simulink model "isi_corr"  (Simulink 7.0)
settings: Solver=ode45  StartTime=0.0  StopTime=500  RelTol=1e-3
undefined symbols (not defined in the file): bit_rev, pfb_coeff_gen_calc
libraries: xbsIndex_r4 x4180, casper_library x399, isi_corr_lib x8, xps_library x5, dspsigops x2, simulink x1
(part 2 of 45)

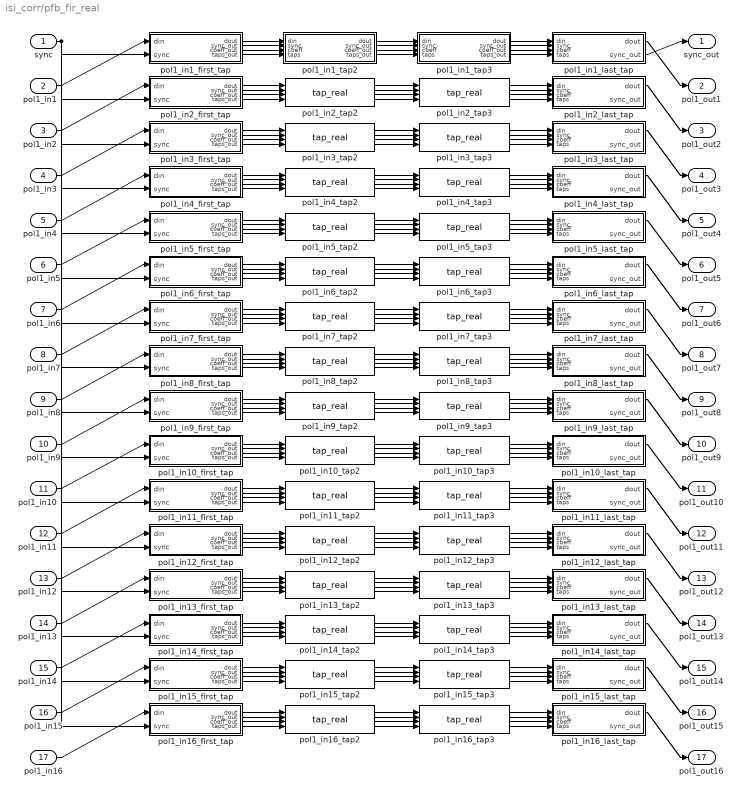
== system isi_corr/pfb_fir_real ==
  sync : Inport  OutDataType=sfix(16)  OutScaling=2^0
  pol1_in1 : Inport  Port=2  OutDataType=sfix(16)  OutScaling=2^0
  pol1_in2 : Inport  Port=3
  pol1_in3 : Inport  Port=4
  pol1_in4 : Inport  Port=5
  pol1_in5 : Inport  Port=6
  pol1_in6 : Inport  Port=7
  pol1_in7 : Inport  Port=8
  pol1_in8 : Inport  Port=9
  pol1_in9 : Inport  Port=10
  pol1_in10 : Inport  Port=11
  pol1_in11 : Inport  Port=12
  pol1_in12 : Inport  Port=13
  pol1_in13 : Inport  Port=14
  pol1_in14 : Inport  Port=15
  pol1_in15 : Inport  Port=16
  pol1_in16 : Inport  Port=17
  pol1_in10_first_tap : Subsystem  AncestorBlock=casper_library/PFBs/first_tap_real  mask(first_tap_real){nput=9, PFBSize=8, CoeffBitWidth=18, TotalTaps=4, BitWidthIn=8, CoeffDistMem=0, WindowType=hamming, mult_latency=2, bram_latency=2, n_inputs=4, fwidth=1}
      mask prompts: nput "This is input number:"; PFBSize "Size of PFB: (2^? pnts)"; CoeffBitWidth "Bitwidth of Coefficients:"; TotalTaps "Total Number of Taps:"; BitWidthIn "Input Bitwidth:"; CoeffDistMem "Implement Coeff Gen in Distributed Memory:"; WindowType "Windowing Function:"; mult_latency "Mult Latency"; bram_latency "BRAM Latency"; n_inputs "Number of Simultaneous Inputs: (2^?)"; fwidth "Bit Width (normal=1)"
      mask init:
      | first_tap_real_init(gcb,...
      |     'nput', nput,...
      |     'PFBSize', PFBSize,...
      |     'CoeffBitWidth', CoeffBitWidth,...
      |     'TotalTaps', TotalTaps,...
      |     'BitWidthIn', BitWidthIn,...
      |     'CoeffDistMem', CoeffDistMem,...
      |     'WindowType', WindowType,...
      |     'mult_latency', mult_latency,...
      |     'bram_latency', bram_latency,...
      |     'n_inputs', n_inputs,...
      |     'fwidth', fwidth);
      settings: AttributesFormatString=input=9, taps=4
      mask StyleString: edit,edit,edit,edit,edit,edit,popup(bartlett|barthannwin|blackman|blackmanharris|bohamwin|chebwin|flattopwin|gausswin|hamming|hann|kaiser|nuttallwin|parzenwin|rectwin|tukeywin|triang|userwindow),edit,edit,edit,edit
  pol1_in10_last_tap : Subsystem  AncestorBlock=casper_library/PFBs/last_tap_real  mask(last_tap_real){TotalTaps=4, BitWidthIn=8, BitWidthOut=18, CoeffBitWidth=18, add_latency=1, mult_latency=2, quantization=Truncate}
      mask prompts: TotalTaps "Total Number of Taps:"; BitWidthIn "Input Bitwidth:"; BitWidthOut "Output Bitwidth:"; CoeffBitWidth "Coeff Bitwidth:"; add_latency "Add Latency"; mult_latency "Mult Latency"; quantization "Quantization Behavior"
      mask init:
      | last_tap_real_init(gcb,...
      |     'TotalTaps', TotalTaps,...
      |     'BitWidthIn', BitWidthIn,...
      |     'BitWidthOut', BitWidthOut,...
      |     'CoeffBitWidth', CoeffBitWidth,...
      |     'add_latency', add_latency,...
      |     'mult_latency', mult_latency,...
      |     'quantization', quantization);
      settings: AttributesFormatString=taps=4
      mask StyleString: edit,edit,edit,edit,edit,edit,popup(Truncate|Round  (unbiased: +/- Inf)|Round  (unbiased: Even Values))
  pol1_in10_tap2 : casper_library/PFBs/tap_real  SystemSampleTime=-1  mult_latency=2  coeff_width=18  coeff_frac_width=17  delay=16  data_width=8  bram_latency=2
  pol1_in10_tap3 : casper_library/PFBs/tap_real  SystemSampleTime=-1  mult_latency=2  coeff_width=18  coeff_frac_width=17  delay=16  data_width=8  bram_latency=2
  pol1_in11_first_tap : Subsystem  AncestorBlock=casper_library/PFBs/first_tap_real  mask(first_tap_real){nput=10, PFBSize=8, CoeffBitWidth=18, TotalTaps=4, BitWidthIn=8, CoeffDistMem=0, WindowType=hamming, mult_latency=2, bram_latency=2, n_inputs=4, fwidth=1}
      mask prompts: nput "This is input number:"; PFBSize "Size of PFB: (2^? pnts)"; CoeffBitWidth "Bitwidth of Coefficients:"; TotalTaps "Total Number of Taps:"; BitWidthIn "Input Bitwidth:"; CoeffDistMem "Implement Coeff Gen in Distributed Memory:"; WindowType "Windowing Function:"; mult_latency "Mult Latency"; bram_latency "BRAM Latency"; n_inputs "Number of Simultaneous Inputs: (2^?)"; fwidth "Bit Width (normal=1)"
      mask init: same as isi_corr/pfb_fir_real/pol1_in10_first_tap
      settings: AttributesFormatString=input=10, taps=4
      mask StyleString: edit,edit,edit,edit,edit,edit,popup(bartlett|barthannwin|blackman|blackmanharris|bohamwin|chebwin|flattopwin|gausswin|hamming|hann|kaiser|nuttallwin|parzenwin|rectwin|tukeywin|triang|userwindow),edit,edit,edit,edit
  pol1_in11_last_tap : Subsystem  AncestorBlock=casper_library/PFBs/last_tap_real  mask(last_tap_real){TotalTaps=4, BitWidthIn=8, BitWidthOut=18, CoeffBitWidth=18, add_latency=1, mult_latency=2, quantization=Truncate}
      mask prompts: TotalTaps "Total Number of Taps:"; BitWidthIn "Input Bitwidth:"; BitWidthOut "Output Bitwidth:"; CoeffBitWidth "Coeff Bitwidth:"; add_latency "Add Latency"; mult_latency "Mult Latency"; quantization "Quantization Behavior"
      mask init: same as isi_corr/pfb_fir_real/pol1_in10_last_tap
      settings: AttributesFormatString=taps=4
      mask StyleString: edit,edit,edit,edit,edit,edit,popup(Truncate|Round  (unbiased: +/- Inf)|Round  (unbiased: Even Values))
  pol1_in11_tap2 : casper_library/PFBs/tap_real  SystemSampleTime=-1  mult_latency=2  coeff_width=18  coeff_frac_width=17  delay=16  data_width=8  bram_latency=2
  pol1_in11_tap3 : casper_library/PFBs/tap_real  SystemSampleTime=-1  mult_latency=2  coeff_width=18  coeff_frac_width=17  delay=16  data_width=8  bram_latency=2
  pol1_in12_first_tap : Subsystem  AncestorBlock=casper_library/PFBs/first_tap_real  mask(first_tap_real){nput=11, PFBSize=8, CoeffBitWidth=18, TotalTaps=4, BitWidthIn=8, CoeffDistMem=0, WindowType=hamming, mult_latency=2, bram_latency=2, n_inputs=4, fwidth=1}
      mask prompts: nput "This is input number:"; PFBSize "Size of PFB: (2^? pnts)"; CoeffBitWidth "Bitwidth of Coefficients:"; TotalTaps "Total Number of Taps:"; BitWidthIn "Input Bitwidth:"; CoeffDistMem "Implement Coeff Gen in Distributed Memory:"; WindowType "Windowing Function:"; mult_latency "Mult Latency"; bram_latency "BRAM Latency"; n_inputs "Number of Simultaneous Inputs: (2^?)"; fwidth "Bit Width (normal=1)"
      mask init: same as isi_corr/pfb_fir_real/pol1_in10_first_tap
      settings: AttributesFormatString=input=11, taps=4
      mask StyleString: edit,edit,edit,edit,edit,edit,popup(bartlett|barthannwin|blackman|blackmanharris|bohamwin|chebwin|flattopwin|gausswin|hamming|hann|kaiser|nuttallwin|parzenwin|rectwin|tukeywin|triang|userwindow),edit,edit,edit,edit
  pol1_in12_last_tap : Subsystem  AncestorBlock=casper_library/PFBs/last_tap_real  mask(last_tap_real){TotalTaps=4, BitWidthIn=8, BitWidthOut=18, CoeffBitWidth=18, add_latency=1, mult_latency=2, quantization=Truncate}
      mask prompts: TotalTaps "Total Number of Taps:"; BitWidthIn "Input Bitwidth:"; BitWidthOut "Output Bitwidth:"; CoeffBitWidth "Coeff Bitwidth:"; add_latency "Add Latency"; mult_latency "Mult Latency"; quantization "Quantization Behavior"
      mask init: same as isi_corr/pfb_fir_real/pol1_in10_last_tap
      settings: AttributesFormatString=taps=4
      mask StyleString: edit,edit,edit,edit,edit,edit,popup(Truncate|Round  (unbiased: +/- Inf)|Round  (unbiased: Even Values))
  pol1_in12_tap2 : casper_library/PFBs/tap_real  SystemSampleTime=-1  mult_latency=2  coeff_width=18  coeff_frac_width=17  delay=16  data_width=8  bram_latency=2
  pol1_in12_tap3 : casper_library/PFBs/tap_real  SystemSampleTime=-1  mult_latency=2  coeff_width=18  coeff_frac_width=17  delay=16  data_width=8  bram_latency=2
  pol1_in13_first_tap : Subsystem  AncestorBlock=casper_library/PFBs/first_tap_real  mask(first_tap_real){nput=12, PFBSize=8, CoeffBitWidth=18, TotalTaps=4, BitWidthIn=8, CoeffDistMem=0, WindowType=hamming, mult_latency=2, bram_latency=2, n_inputs=4, fwidth=1}
      mask prompts: nput "This is input number:"; PFBSize "Size of PFB: (2^? pnts)"; CoeffBitWidth "Bitwidth of Coefficients:"; TotalTaps "Total Number of Taps:"; BitWidthIn "Input Bitwidth:"; CoeffDistMem "Implement Coeff Gen in Distributed Memory:"; WindowType "Windowing Function:"; mult_latency "Mult Latency"; bram_latency "BRAM Latency"; n_inputs "Number of Simultaneous Inputs: (2^?)"; fwidth "Bit Width (normal=1)"
      mask init: same as isi_corr/pfb_fir_real/pol1_in10_first_tap
      settings: AttributesFormatString=input=12, taps=4
      mask StyleString: edit,edit,edit,edit,edit,edit,popup(bartlett|barthannwin|blackman|blackmanharris|bohamwin|chebwin|flattopwin|gausswin|hamming|hann|kaiser|nuttallwin|parzenwin|rectwin|tukeywin|triang|userwindow),edit,edit,edit,edit
  pol1_in13_last_tap : Subsystem  AncestorBlock=casper_library/PFBs/last_tap_real  mask(last_tap_real){TotalTaps=4, BitWidthIn=8, BitWidthOut=18, CoeffBitWidth=18, add_latency=1, mult_latency=2, quantization=Truncate}
      mask prompts: TotalTaps "Total Number of Taps:"; BitWidthIn "Input Bitwidth:"; BitWidthOut "Output Bitwidth:"; CoeffBitWidth "Coeff Bitwidth:"; add_latency "Add Latency"; mult_latency "Mult Latency"; quantization "Quantization Behavior"
      mask init: same as isi_corr/pfb_fir_real/pol1_in10_last_tap
      settings: AttributesFormatString=taps=4
      mask StyleString: edit,edit,edit,edit,edit,edit,popup(Truncate|Round  (unbiased: +/- Inf)|Round  (unbiased: Even Values))
  pol1_in13_tap2 : casper_library/PFBs/tap_real  SystemSampleTime=-1  mult_latency=2  coeff_width=18  coeff_frac_width=17  delay=16  data_width=8  bram_latency=2
  pol1_in13_tap3 : casper_library/PFBs/tap_real  SystemSampleTime=-1  mult_latency=2  coeff_width=18  coeff_frac_width=17  delay=16  data_width=8  bram_latency=2
  pol1_in14_first_tap : Subsystem  AncestorBlock=casper_library/PFBs/first_tap_real  mask(first_tap_real){nput=13, PFBSize=8, CoeffBitWidth=18, TotalTaps=4, BitWidthIn=8, CoeffDistMem=0, WindowType=hamming, mult_latency=2, bram_latency=2, n_inputs=4, fwidth=1}
      mask prompts: nput "This is input number:"; PFBSize "Size of PFB: (2^? pnts)"; CoeffBitWidth "Bitwidth of Coefficients:"; TotalTaps "Total Number of Taps:"; BitWidthIn "Input Bitwidth:"; CoeffDistMem "Implement Coeff Gen in Distributed Memory:"; WindowType "Windowing Function:"; mult_latency "Mult Latency"; bram_latency "BRAM Latency"; n_inputs "Number of Simultaneous Inputs: (2^?)"; fwidth "Bit Width (normal=1)"
      mask init: same as isi_corr/pfb_fir_real/pol1_in10_first_tap
      settings: AttributesFormatString=input=13, taps=4
      mask StyleString: edit,edit,edit,edit,edit,edit,popup(bartlett|barthannwin|blackman|blackmanharris|bohamwin|chebwin|flattopwin|gausswin|hamming|hann|kaiser|nuttallwin|parzenwin|rectwin|tukeywin|triang|userwindow),edit,edit,edit,edit
  pol1_in14_last_tap : Subsystem  AncestorBlock=casper_library/PFBs/last_tap_real  mask(last_tap_real){TotalTaps=4, BitWidthIn=8, BitWidthOut=18, CoeffBitWidth=18, add_latency=1, mult_latency=2, quantization=Truncate}
      mask prompts: TotalTaps "Total Number of Taps:"; BitWidthIn "Input Bitwidth:"; BitWidthOut "Output Bitwidth:"; CoeffBitWidth "Coeff Bitwidth:"; add_latency "Add Latency"; mult_latency "Mult Latency"; quantization "Quantization Behavior"
      mask init: same as isi_corr/pfb_fir_real/pol1_in10_last_tap
      settings: AttributesFormatString=taps=4
      mask StyleString: edit,edit,edit,edit,edit,edit,popup(Truncate|Round  (unbiased: +/- Inf)|Round  (unbiased: Even Values))
  pol1_in14_tap2 : casper_library/PFBs/tap_real  SystemSampleTime=-1  mult_latency=2  coeff_width=18  coeff_frac_width=17  delay=16  data_width=8  bram_latency=2
  pol1_in14_tap3 : casper_library/PFBs/tap_real  SystemSampleTime=-1  mult_latency=2  coeff_width=18  coeff_frac_width=17  delay=16  data_width=8  bram_latency=2
  pol1_in15_first_tap : Subsystem  AncestorBlock=casper_library/PFBs/first_tap_real  mask(first_tap_real){nput=14, PFBSize=8, CoeffBitWidth=18, TotalTaps=4, BitWidthIn=8, CoeffDistMem=0, WindowType=hamming, mult_latency=2, bram_latency=2, n_inputs=4, fwidth=1}
      mask prompts: nput "This is input number:"; PFBSize "Size of PFB: (2^? pnts)"; CoeffBitWidth "Bitwidth of Coefficients:"; TotalTaps "Total Number of Taps:"; BitWidthIn "Input Bitwidth:"; CoeffDistMem "Implement Coeff Gen in Distributed Memory:"; WindowType "Windowing Function:"; mult_latency "Mult Latency"; bram_latency "BRAM Latency"; n_inputs "Number of Simultaneous Inputs: (2^?)"; fwidth "Bit Width (normal=1)"
      mask init: same as isi_corr/pfb_fir_real/pol1_in10_first_tap
      settings: AttributesFormatString=input=14, taps=4
      mask StyleString: edit,edit,edit,edit,edit,edit,popup(bartlett|barthannwin|blackman|blackmanharris|bohamwin|chebwin|flattopwin|gausswin|hamming|hann|kaiser|nuttallwin|parzenwin|rectwin|tukeywin|triang|userwindow),edit,edit,edit,edit
  pol1_in15_last_tap : Subsystem  AncestorBlock=casper_library/PFBs/last_tap_real  mask(last_tap_real){TotalTaps=4, BitWidthIn=8, BitWidthOut=18, CoeffBitWidth=18, add_latency=1, mult_latency=2, quantization=Truncate}
      mask prompts: TotalTaps "Total Number of Taps:"; BitWidthIn "Input Bitwidth:"; BitWidthOut "Output Bitwidth:"; CoeffBitWidth "Coeff Bitwidth:"; add_latency "Add Latency"; mult_latency "Mult Latency"; quantization "Quantization Behavior"
      mask init: same as isi_corr/pfb_fir_real/pol1_in10_last_tap
      settings: AttributesFormatString=taps=4
      mask StyleString: edit,edit,edit,edit,edit,edit,popup(Truncate|Round  (unbiased: +/- Inf)|Round  (unbiased: Even Values))
  pol1_in15_tap2 : casper_library/PFBs/tap_real  SystemSampleTime=-1  mult_latency=2  coeff_width=18  coeff_frac_width=17  delay=16  data_width=8  bram_latency=2
  pol1_in15_tap3 : casper_library/PFBs/tap_real  SystemSampleTime=-1  mult_latency=2  coeff_width=18  coeff_frac_width=17  delay=16  data_width=8  bram_latency=2
  pol1_in16_first_tap : Subsystem  AncestorBlock=casper_library/PFBs/first_tap_real  mask(first_tap_real){nput=15, PFBSize=8, CoeffBitWidth=18, TotalTaps=4, BitWidthIn=8, CoeffDistMem=0, WindowType=hamming, mult_latency=2, bram_latency=2, n_inputs=4, fwidth=1}
      mask prompts: nput "This is input number:"; PFBSize "Size of PFB: (2^? pnts)"; CoeffBitWidth "Bitwidth of Coefficients:"; TotalTaps "Total Number of Taps:"; BitWidthIn "Input Bitwidth:"; CoeffDistMem "Implement Coeff Gen in Distributed Memory:"; WindowType "Windowing Function:"; mult_latency "Mult Latency"; bram_latency "BRAM Latency"; n_inputs "Number of Simultaneous Inputs: (2^?)"; fwidth "Bit Width (normal=1)"
      mask init: same as isi_corr/pfb_fir_real/pol1_in10_first_tap
      settings: AttributesFormatString=input=15, taps=4
      mask StyleString: edit,edit,edit,edit,edit,edit,popup(bartlett|barthannwin|blackman|blackmanharris|bohamwin|chebwin|flattopwin|gausswin|hamming|hann|kaiser|nuttallwin|parzenwin|rectwin|tukeywin|triang|userwindow),edit,edit,edit,edit
  pol1_in16_last_tap : Subsystem  AncestorBlock=casper_library/PFBs/last_tap_real  mask(last_tap_real){TotalTaps=4, BitWidthIn=8, BitWidthOut=18, CoeffBitWidth=18, add_latency=1, mult_latency=2, quantization=Truncate}
      mask prompts: TotalTaps "Total Number of Taps:"; BitWidthIn "Input Bitwidth:"; BitWidthOut "Output Bitwidth:"; CoeffBitWidth "Coeff Bitwidth:"; add_latency "Add Latency"; mult_latency "Mult Latency"; quantization "Quantization Behavior"
      mask init: same as isi_corr/pfb_fir_real/pol1_in10_last_tap
      settings: AttributesFormatString=taps=4
      mask StyleString: edit,edit,edit,edit,edit,edit,popup(Truncate|Round  (unbiased: +/- Inf)|Round  (unbiased: Even Values))
  pol1_in16_tap2 : casper_library/PFBs/tap_real  SystemSampleTime=-1  mult_latency=2  coeff_width=18  coeff_frac_width=17  delay=16  data_width=8  bram_latency=2
  pol1_in16_tap3 : casper_library/PFBs/tap_real  SystemSampleTime=-1  mult_latency=2  coeff_width=18  coeff_frac_width=17  delay=16  data_width=8  bram_latency=2
  pol1_in1_first_tap : Subsystem  AncestorBlock=casper_library/PFBs/first_tap_real  mask(first_tap_real){nput=0, PFBSize=8, CoeffBitWidth=18, TotalTaps=4, BitWidthIn=8, CoeffDistMem=0, WindowType=hamming, mult_latency=2, bram_latency=2, n_inputs=4, fwidth=1}
      mask prompts: nput "This is input number:"; PFBSize "Size of PFB: (2^? pnts)"; CoeffBitWidth "Bitwidth of Coefficients:"; TotalTaps "Total Number of Taps:"; BitWidthIn "Input Bitwidth:"; CoeffDistMem "Implement Coeff Gen in Distributed Memory:"; WindowType "Windowing Function:"; mult_latency "Mult Latency"; bram_latency "BRAM Latency"; n_inputs "Number of Simultaneous Inputs: (2^?)"; fwidth "Bit Width (normal=1)"
      mask init: same as isi_corr/pfb_fir_real/pol1_in10_first_tap
      settings: AttributesFormatString=input=0, taps=4
      mask StyleString: edit,edit,edit,edit,edit,edit,popup(bartlett|barthannwin|blackman|blackmanharris|bohamwin|chebwin|flattopwin|gausswin|hamming|hann|kaiser|nuttallwin|parzenwin|rectwin|tukeywin|triang|userwindow),edit,edit,edit,edit
  pol1_in1_last_tap : Subsystem  AncestorBlock=casper_library/PFBs/last_tap_real  mask(last_tap_real){TotalTaps=4, BitWidthIn=8, BitWidthOut=18, CoeffBitWidth=18, add_latency=1, mult_latency=2, quantization=Truncate}
      mask prompts: TotalTaps "Total Number of Taps:"; BitWidthIn "Input Bitwidth:"; BitWidthOut "Output Bitwidth:"; CoeffBitWidth "Coeff Bitwidth:"; add_latency "Add Latency"; mult_latency "Mult Latency"; quantization "Quantization Behavior"
      mask init: same as isi_corr/pfb_fir_real/pol1_in10_last_tap
      settings: AttributesFormatString=taps=4
      mask StyleString: edit,edit,edit,edit,edit,edit,popup(Truncate|Round  (unbiased: +/- Inf)|Round  (unbiased: Even Values))
  pol1_in1_tap2 : Subsystem  AncestorBlock=casper_library/PFBs/tap_real  mask(pfb_tap_real){mult_latency=2, coeff_width=18, coeff_frac_width=17, delay=16, data_width=8, bram_latency=2}
      mask prompts: mult_latency "Multiplier Latency"; coeff_width "Coefficient Bit Width In"; coeff_frac_width "Coefficient Fractional Bits"; delay "Data/Sync Delay"; data_width "Data Bit Width In"; bram_latency "BRAM Latency"
      mask init:
      | cursys = gcb;
      | disp(cursys);
  pol1_in1_tap3 : Subsystem  AncestorBlock=casper_library/PFBs/tap_real  mask(pfb_tap_real){mult_latency=2, coeff_width=18, coeff_frac_width=17, delay=16, data_width=8, bram_latency=2}
      mask prompts: mult_latency "Multiplier Latency"; coeff_width "Coefficient Bit Width In"; coeff_frac_width "Coefficient Fractional Bits"; delay "Data/Sync Delay"; data_width "Data Bit Width In"; bram_latency "BRAM Latency"
      mask init:
      | cursys = gcb;
      | disp(cursys);
  pol1_in2_first_tap : Subsystem  AncestorBlock=casper_library/PFBs/first_tap_real  mask(first_tap_real){nput=1, PFBSize=8, CoeffBitWidth=18, TotalTaps=4, BitWidthIn=8, CoeffDistMem=0, WindowType=hamming, mult_latency=2, bram_latency=2, n_inputs=4, fwidth=1}
      mask prompts: nput "This is input number:"; PFBSize "Size of PFB: (2^? pnts)"; CoeffBitWidth "Bitwidth of Coefficients:"; TotalTaps "Total Number of Taps:"; BitWidthIn "Input Bitwidth:"; CoeffDistMem "Implement Coeff Gen in Distributed Memory:"; WindowType "Windowing Function:"; mult_latency "Mult Latency"; bram_latency "BRAM Latency"; n_inputs "Number of Simultaneous Inputs: (2^?)"; fwidth "Bit Width (normal=1)"
      mask init: same as isi_corr/pfb_fir_real/pol1_in10_first_tap
      settings: AttributesFormatString=input=1, taps=4
      mask StyleString: edit,edit,edit,edit,edit,edit,popup(bartlett|barthannwin|blackman|blackmanharris|bohamwin|chebwin|flattopwin|gausswin|hamming|hann|kaiser|nuttallwin|parzenwin|rectwin|tukeywin|triang|userwindow),edit,edit,edit,edit
  pol1_in2_last_tap : Subsystem  AncestorBlock=casper_library/PFBs/last_tap_real  mask(last_tap_real){TotalTaps=4, BitWidthIn=8, BitWidthOut=18, CoeffBitWidth=18, add_latency=1, mult_latency=2, quantization=Truncate}
      mask prompts: TotalTaps "Total Number of Taps:"; BitWidthIn "Input Bitwidth:"; BitWidthOut "Output Bitwidth:"; CoeffBitWidth "Coeff Bitwidth:"; add_latency "Add Latency"; mult_latency "Mult Latency"; quantization "Quantization Behavior"
      mask init: same as isi_corr/pfb_fir_real/pol1_in10_last_tap
      settings: AttributesFormatString=taps=4
      mask StyleString: edit,edit,edit,edit,edit,edit,popup(Truncate|Round  (unbiased: +/- Inf)|Round  (unbiased: Even Values))
  pol1_in2_tap2 : casper_library/PFBs/tap_real  SystemSampleTime=-1  mult_latency=2  coeff_width=18  coeff_frac_width=17  delay=16  data_width=8  bram_latency=2
  pol1_in2_tap3 : casper_library/PFBs/tap_real  SystemSampleTime=-1  mult_latency=2  coeff_width=18  coeff_frac_width=17  delay=16  data_width=8  bram_latency=2
  pol1_in3_first_tap : Subsystem  AncestorBlock=casper_library/PFBs/first_tap_real  mask(first_tap_real){nput=2, PFBSize=8, CoeffBitWidth=18, TotalTaps=4, BitWidthIn=8, CoeffDistMem=0, WindowType=hamming, mult_latency=2, bram_latency=2, n_inputs=4, fwidth=1}
      mask prompts: nput "This is input number:"; PFBSize "Size of PFB: (2^? pnts)"; CoeffBitWidth "Bitwidth of Coefficients:"; TotalTaps "Total Number of Taps:"; BitWidthIn "Input Bitwidth:"; CoeffDistMem "Implement Coeff Gen in Distributed Memory:"; WindowType "Windowing Function:"; mult_latency "Mult Latency"; bram_latency "BRAM Latency"; n_inputs "Number of Simultaneous Inputs: (2^?)"; fwidth "Bit Width (normal=1)"
      mask init: same as isi_corr/pfb_fir_real/pol1_in10_first_tap
      settings: AttributesFormatString=input=2, taps=4
      mask StyleString: edit,edit,edit,edit,edit,edit,popup(bartlett|barthannwin|blackman|blackmanharris|bohamwin|chebwin|flattopwin|gausswin|hamming|hann|kaiser|nuttallwin|parzenwin|rectwin|tukeywin|triang|userwindow),edit,edit,edit,edit
  pol1_in3_last_tap : Subsystem  AncestorBlock=casper_library/PFBs/last_tap_real  mask(last_tap_real){TotalTaps=4, BitWidthIn=8, BitWidthOut=18, CoeffBitWidth=18, add_latency=1, mult_latency=2, quantization=Truncate}
      mask prompts: TotalTaps "Total Number of Taps:"; BitWidthIn "Input Bitwidth:"; BitWidthOut "Output Bitwidth:"; CoeffBitWidth "Coeff Bitwidth:"; add_latency "Add Latency"; mult_latency "Mult Latency"; quantization "Quantization Behavior"
      mask init: same as isi_corr/pfb_fir_real/pol1_in10_last_tap
      settings: AttributesFormatString=taps=4
      mask StyleString: edit,edit,edit,edit,edit,edit,popup(Truncate|Round  (unbiased: +/- Inf)|Round  (unbiased: Even Values))
  pol1_in3_tap2 : casper_library/PFBs/tap_real  SystemSampleTime=-1  mult_latency=2  coeff_width=18  coeff_frac_width=17  delay=16  data_width=8  bram_latency=2
  pol1_in3_tap3 : casper_library/PFBs/tap_real  SystemSampleTime=-1  mult_latency=2  coeff_width=18  coeff_frac_width=17  delay=16  data_width=8  bram_latency=2
  pol1_in4_first_tap : Subsystem  AncestorBlock=casper_library/PFBs/first_tap_real  mask(first_tap_real){nput=3, PFBSize=8, CoeffBitWidth=18, TotalTaps=4, BitWidthIn=8, CoeffDistMem=0, WindowType=hamming, mult_latency=2, bram_latency=2, n_inputs=4, fwidth=1}
      mask prompts: nput "This is input number:"; PFBSize "Size of PFB: (2^? pnts)"; CoeffBitWidth "Bitwidth of Coefficients:"; TotalTaps "Total Number of Taps:"; BitWidthIn "Input Bitwidth:"; CoeffDistMem "Implement Coeff Gen in Distributed Memory:"; WindowType "Windowing Function:"; mult_latency "Mult Latency"; bram_latency "BRAM Latency"; n_inputs "Number of Simultaneous Inputs: (2^?)"; fwidth "Bit Width (normal=1)"
      mask init: same as isi_corr/pfb_fir_real/pol1_in10_first_tap
      settings: AttributesFormatString=input=3, taps=4
      mask StyleString: edit,edit,edit,edit,edit,edit,popup(bartlett|barthannwin|blackman|blackmanharris|bohamwin|chebwin|flattopwin|gausswin|hamming|hann|kaiser|nuttallwin|parzenwin|rectwin|tukeywin|triang|userwindow),edit,edit,edit,edit
  pol1_in4_last_tap : Subsystem  AncestorBlock=casper_library/PFBs/last_tap_real  mask(last_tap_real){TotalTaps=4, BitWidthIn=8, BitWidthOut=18, CoeffBitWidth=18, add_latency=1, mult_latency=2, quantization=Truncate}
      mask prompts: TotalTaps "Total Number of Taps:"; BitWidthIn "Input Bitwidth:"; BitWidthOut "Output Bitwidth:"; CoeffBitWidth "Coeff Bitwidth:"; add_latency "Add Latency"; mult_latency "Mult Latency"; quantization "Quantization Behavior"
      mask init: same as isi_corr/pfb_fir_real/pol1_in10_last_tap
      settings: AttributesFormatString=taps=4
      mask StyleString: edit,edit,edit,edit,edit,edit,popup(Truncate|Round  (unbiased: +/- Inf)|Round  (unbiased: Even Values))
  pol1_in4_tap2 : casper_library/PFBs/tap_real  SystemSampleTime=-1  mult_latency=2  coeff_width=18  coeff_frac_width=17  delay=16  data_width=8  bram_latency=2
  pol1_in4_tap3 : casper_library/PFBs/tap_real  SystemSampleTime=-1  mult_latency=2  coeff_width=18  coeff_frac_width=17  delay=16  data_width=8  bram_latency=2
  pol1_in5_first_tap : Subsystem  AncestorBlock=casper_library/PFBs/first_tap_real  mask(first_tap_real){nput=4, PFBSize=8, CoeffBitWidth=18, TotalTaps=4, BitWidthIn=8, CoeffDistMem=0, WindowType=hamming, mult_latency=2, bram_latency=2, n_inputs=4, fwidth=1}
      mask prompts: nput "This is input number:"; PFBSize "Size of PFB: (2^? pnts)"; CoeffBitWidth "Bitwidth of Coefficients:"; TotalTaps "Total Number of Taps:"; BitWidthIn "Input Bitwidth:"; CoeffDistMem "Implement Coeff Gen in Distributed Memory:"; WindowType "Windowing Function:"; mult_latency "Mult Latency"; bram_latency "BRAM Latency"; n_inputs "Number of Simultaneous Inputs: (2^?)"; fwidth "Bit Width (normal=1)"
      mask init: same as isi_corr/pfb_fir_real/pol1_in10_first_tap
      settings: AttributesFormatString=input=4, taps=4
      mask StyleString: edit,edit,edit,edit,edit,edit,popup(bartlett|barthannwin|blackman|blackmanharris|bohamwin|chebwin|flattopwin|gausswin|hamming|hann|kaiser|nuttallwin|parzenwin|rectwin|tukeywin|triang|userwindow),edit,edit,edit,edit
  pol1_in5_last_tap : Subsystem  AncestorBlock=casper_library/PFBs/last_tap_real  mask(last_tap_real){TotalTaps=4, BitWidthIn=8, BitWidthOut=18, CoeffBitWidth=18, add_latency=1, mult_latency=2, quantization=Truncate}
      mask prompts: TotalTaps "Total Number of Taps:"; BitWidthIn "Input Bitwidth:"; BitWidthOut "Output Bitwidth:"; CoeffBitWidth "Coeff Bitwidth:"; add_latency "Add Latency"; mult_latency "Mult Latency"; quantization "Quantization Behavior"
      mask init: same as isi_corr/pfb_fir_real/pol1_in10_last_tap
      settings: AttributesFormatString=taps=4
      mask StyleString: edit,edit,edit,edit,edit,edit,popup(Truncate|Round  (unbiased: +/- Inf)|Round  (unbiased: Even Values))
  pol1_in5_tap2 : casper_library/PFBs/tap_real  SystemSampleTime=-1  mult_latency=2  coeff_width=18  coeff_frac_width=17  delay=16  data_width=8  bram_latency=2
  pol1_in5_tap3 : casper_library/PFBs/tap_real  SystemSampleTime=-1  mult_latency=2  coeff_width=18  coeff_frac_width=17  delay=16  data_width=8  bram_latency=2
  pol1_in6_first_tap : Subsystem  AncestorBlock=casper_library/PFBs/first_tap_real  mask(first_tap_real){nput=5, PFBSize=8, CoeffBitWidth=18, TotalTaps=4, BitWidthIn=8, CoeffDistMem=0, WindowType=hamming, mult_latency=2, bram_latency=2, n_inputs=4, fwidth=1}
      mask prompts: nput "This is input number:"; PFBSize "Size of PFB: (2^? pnts)"; CoeffBitWidth "Bitwidth of Coefficients:"; TotalTaps "Total Number of Taps:"; BitWidthIn "Input Bitwidth:"; CoeffDistMem "Implement Coeff Gen in Distributed Memory:"; WindowType "Windowing Function:"; mult_latency "Mult Latency"; bram_latency "BRAM Latency"; n_inputs "Number of Simultaneous Inputs: (2^?)"; fwidth "Bit Width (normal=1)"
      mask init: same as isi_corr/pfb_fir_real/pol1_in10_first_tap
      settings: AttributesFormatString=input=5, taps=4
      mask StyleString: edit,edit,edit,edit,edit,edit,popup(bartlett|barthannwin|blackman|blackmanharris|bohamwin|chebwin|flattopwin|gausswin|hamming|hann|kaiser|nuttallwin|parzenwin|rectwin|tukeywin|triang|userwindow),edit,edit,edit,edit
  pol1_in6_last_tap : Subsystem  AncestorBlock=casper_library/PFBs/last_tap_real  mask(last_tap_real){TotalTaps=4, BitWidthIn=8, BitWidthOut=18, CoeffBitWidth=18, add_latency=1, mult_latency=2, quantization=Truncate}
      mask prompts: TotalTaps "Total Number of Taps:"; BitWidthIn "Input Bitwidth:"; BitWidthOut "Output Bitwidth:"; CoeffBitWidth "Coeff Bitwidth:"; add_latency "Add Latency"; mult_latency "Mult Latency"; quantization "Quantization Behavior"
      mask init: same as isi_corr/pfb_fir_real/pol1_in10_last_tap
      settings: AttributesFormatString=taps=4
      mask StyleString: edit,edit,edit,edit,edit,edit,popup(Truncate|Round  (unbiased: +/- Inf)|Round  (unbiased: Even Values))
  pol1_in6_tap2 : casper_library/PFBs/tap_real  SystemSampleTime=-1  mult_latency=2  coeff_width=18  coeff_frac_width=17  delay=16  data_width=8  bram_latency=2
  pol1_in6_tap3 : casper_library/PFBs/tap_real  SystemSampleTime=-1  mult_latency=2  coeff_width=18  coeff_frac_width=17  delay=16  data_width=8  bram_latency=2
  pol1_in7_first_tap : Subsystem  AncestorBlock=casper_library/PFBs/first_tap_real  mask(first_tap_real){nput=6, PFBSize=8, CoeffBitWidth=18, TotalTaps=4, BitWidthIn=8, CoeffDistMem=0, WindowType=hamming, mult_latency=2, bram_latency=2, n_inputs=4, fwidth=1}
      mask prompts: nput "This is input number:"; PFBSize "Size of PFB: (2^? pnts)"; CoeffBitWidth "Bitwidth of Coefficients:"; TotalTaps "Total Number of Taps:"; BitWidthIn "Input Bitwidth:"; CoeffDistMem "Implement Coeff Gen in Distributed Memory:"; WindowType "Windowing Function:"; mult_latency "Mult Latency"; bram_latency "BRAM Latency"; n_inputs "Number of Simultaneous Inputs: (2^?)"; fwidth "Bit Width (normal=1)"
      mask init: same as isi_corr/pfb_fir_real/pol1_in10_first_tap
      settings: AttributesFormatString=input=6, taps=4
      mask StyleString: edit,edit,edit,edit,edit,edit,popup(bartlett|barthannwin|blackman|blackmanharris|bohamwin|chebwin|flattopwin|gausswin|hamming|hann|kaiser|nuttallwin|parzenwin|rectwin|tukeywin|triang|userwindow),edit,edit,edit,edit
  pol1_in7_last_tap : Subsystem  AncestorBlock=casper_library/PFBs/last_tap_real  mask(last_tap_real){TotalTaps=4, BitWidthIn=8, BitWidthOut=18, CoeffBitWidth=18, add_latency=1, mult_latency=2, quantization=Truncate}
      mask prompts: TotalTaps "Total Number of Taps:"; BitWidthIn "Input Bitwidth:"; BitWidthOut "Output Bitwidth:"; CoeffBitWidth "Coeff Bitwidth:"; add_latency "Add Latency"; mult_latency "Mult Latency"; quantization "Quantization Behavior"
      mask init: same as isi_corr/pfb_fir_real/pol1_in10_last_tap
      settings: AttributesFormatString=taps=4
      mask StyleString: edit,edit,edit,edit,edit,edit,popup(Truncate|Round  (unbiased: +/- Inf)|Round  (unbiased: Even Values))
  pol1_in7_tap2 : casper_library/PFBs/tap_real  SystemSampleTime=-1  mult_latency=2  coeff_width=18  coeff_frac_width=17  delay=16  data_width=8  bram_latency=2
  pol1_in7_tap3 : casper_library/PFBs/tap_real  SystemSampleTime=-1  mult_latency=2  coeff_width=18  coeff_frac_width=17  delay=16  data_width=8  bram_latency=2
  pol1_in8_first_tap : Subsystem  AncestorBlock=casper_library/PFBs/first_tap_real  mask(first_tap_real){nput=7, PFBSize=8, CoeffBitWidth=18, TotalTaps=4, BitWidthIn=8, CoeffDistMem=0, WindowType=hamming, mult_latency=2, bram_latency=2, n_inputs=4, fwidth=1}
      mask prompts: nput "This is input number:"; PFBSize "Size of PFB: (2^? pnts)"; CoeffBitWidth "Bitwidth of Coefficients:"; TotalTaps "Total Number of Taps:"; BitWidthIn "Input Bitwidth:"; CoeffDistMem "Implement Coeff Gen in Distributed Memory:"; WindowType "Windowing Function:"; mult_latency "Mult Latency"; bram_latency "BRAM Latency"; n_inputs "Number of Simultaneous Inputs: (2^?)"; fwidth "Bit Width (normal=1)"
      mask init: same as isi_corr/pfb_fir_real/pol1_in10_first_tap
      settings: AttributesFormatString=input=7, taps=4
      mask StyleString: edit,edit,edit,edit,edit,edit,popup(bartlett|barthannwin|blackman|blackmanharris|bohamwin|chebwin|flattopwin|gausswin|hamming|hann|kaiser|nuttallwin|parzenwin|rectwin|tukeywin|triang|userwindow),edit,edit,edit,edit
  pol1_in8_last_tap : Subsystem  AncestorBlock=casper_library/PFBs/last_tap_real  mask(last_tap_real){TotalTaps=4, BitWidthIn=8, BitWidthOut=18, CoeffBitWidth=18, add_latency=1, mult_latency=2, quantization=Truncate}
      mask prompts: TotalTaps "Total Number of Taps:"; BitWidthIn "Input Bitwidth:"; BitWidthOut "Output Bitwidth:"; CoeffBitWidth "Coeff Bitwidth:"; add_latency "Add Latency"; mult_latency "Mult Latency"; quantization "Quantization Behavior"
      mask init: same as isi_corr/pfb_fir_real/pol1_in10_last_tap
      settings: AttributesFormatString=taps=4
      mask StyleString: edit,edit,edit,edit,edit,edit,popup(Truncate|Round  (unbiased: +/- Inf)|Round  (unbiased: Even Values))
  pol1_in8_tap2 : casper_library/PFBs/tap_real  SystemSampleTime=-1  mult_latency=2  coeff_width=18  coeff_frac_width=17  delay=16  data_width=8  bram_latency=2
  pol1_in8_tap3 : casper_library/PFBs/tap_real  SystemSampleTime=-1  mult_latency=2  coeff_width=18  coeff_frac_width=17  delay=16  data_width=8  bram_latency=2
  pol1_in9_first_tap : Subsystem  AncestorBlock=casper_library/PFBs/first_tap_real  mask(first_tap_real){nput=8, PFBSize=8, CoeffBitWidth=18, TotalTaps=4, BitWidthIn=8, CoeffDistMem=0, WindowType=hamming, mult_latency=2, bram_latency=2, n_inputs=4, fwidth=1}
      mask prompts: nput "This is input number:"; PFBSize "Size of PFB: (2^? pnts)"; CoeffBitWidth "Bitwidth of Coefficients:"; TotalTaps "Total Number of Taps:"; BitWidthIn "Input Bitwidth:"; CoeffDistMem "Implement Coeff Gen in Distributed Memory:"; WindowType "Windowing Function:"; mult_latency "Mult Latency"; bram_latency "BRAM Latency"; n_inputs "Number of Simultaneous Inputs: (2^?)"; fwidth "Bit Width (normal=1)"
      mask init: same as isi_corr/pfb_fir_real/pol1_in10_first_tap
      settings: AttributesFormatString=input=8, taps=4
      mask StyleString: edit,edit,edit,edit,edit,edit,popup(bartlett|barthannwin|blackman|blackmanharris|bohamwin|chebwin|flattopwin|gausswin|hamming|hann|kaiser|nuttallwin|parzenwin|rectwin|tukeywin|triang|userwindow),edit,edit,edit,edit
  pol1_in9_last_tap : Subsystem  AncestorBlock=casper_library/PFBs/last_tap_real  mask(last_tap_real){TotalTaps=4, BitWidthIn=8, BitWidthOut=18, CoeffBitWidth=18, add_latency=1, mult_latency=2, quantization=Truncate}
      mask prompts: TotalTaps "Total Number of Taps:"; BitWidthIn "Input Bitwidth:"; BitWidthOut "Output Bitwidth:"; CoeffBitWidth "Coeff Bitwidth:"; add_latency "Add Latency"; mult_latency "Mult Latency"; quantization "Quantization Behavior"
      mask init: same as isi_corr/pfb_fir_real/pol1_in10_last_tap
      settings: AttributesFormatString=taps=4
      mask StyleString: edit,edit,edit,edit,edit,edit,popup(Truncate|Round  (unbiased: +/- Inf)|Round  (unbiased: Even Values))
  pol1_in9_tap2 : casper_library/PFBs/tap_real  SystemSampleTime=-1  mult_latency=2  coeff_width=18  coeff_frac_width=17  delay=16  data_width=8  bram_latency=2
  pol1_in9_tap3 : casper_library/PFBs/tap_real  SystemSampleTime=-1  mult_latency=2  coeff_width=18  coeff_frac_width=17  delay=16  data_width=8  bram_latency=2
  sync_out : Outport  OutDataType=sfix(16)  OutScaling=2^0
  pol1_out1 : Outport  Port=2  OutDataType=sfix(16)  OutScaling=2^0
  pol1_out2 : Outport  Port=3
  pol1_out3 : Outport  Port=4
  pol1_out4 : Outport  Port=5
  pol1_out5 : Outport  Port=6
  pol1_out6 : Outport  Port=7
  pol1_out7 : Outport  Port=8
  pol1_out8 : Outport  Port=9
  pol1_out9 : Outport  Port=10
  pol1_out10 : Outport  Port=11
  pol1_out11 : Outport  Port=12
  pol1_out12 : Outport  Port=13
  pol1_out13 : Outport  Port=14
  pol1_out14 : Outport  Port=15
  pol1_out15 : Outport  Port=16
  pol1_out16 : Outport  Port=17
  wires:
  pol1_in1/1 -> pol1_in1_first_tap/1(din)
  sync/1 -> pol1_in1_first_tap/2(sync), pol1_in2_first_tap/2(sync), pol1_in3_first_tap/2(sync), pol1_in4_first_tap/2(sync), pol1_in5_first_tap/2(sync), pol1_in6_first_tap/2(sync), pol1_in7_first_tap/2(sync), pol1_in8_first_tap/2(sync), pol1_in9_first_tap/2(sync), pol1_in10_first_tap/2(sync), pol1_in11_first_tap/2(sync), pol1_in12_first_tap/2(sync), pol1_in13_first_tap/2(sync), pol1_in14_first_tap/2(sync), pol1_in15_first_tap/2(sync), pol1_in16_first_tap/2(sync)
  pol1_in1_first_tap/1(dout) -> pol1_in1_tap2/1(din)
  pol1_in1_first_tap/2(sync_out) -> pol1_in1_tap2/2(sync)
  pol1_in1_first_tap/3(coeff_out) -> pol1_in1_tap2/3(coeff)
  pol1_in1_first_tap/4(taps_out) -> pol1_in1_tap2/4(taps)
  pol1_in1_tap2/1(dout) -> pol1_in1_tap3/1(din)
  pol1_in1_tap2/2(sync_out) -> pol1_in1_tap3/2(sync)
  pol1_in1_tap2/3(coeff_out) -> pol1_in1_tap3/3(coeff)
  pol1_in1_tap2/4(taps_out) -> pol1_in1_tap3/4(taps)
  pol1_in1_tap3/1(dout) -> pol1_in1_last_tap/1(din)
  pol1_in1_tap3/2(sync_out) -> pol1_in1_last_tap/2(sync)
  pol1_in1_tap3/3(coeff_out) -> pol1_in1_last_tap/3(coeff)
  pol1_in1_tap3/4(taps_out) -> pol1_in1_last_tap/4(taps)
  pol1_in1_last_tap/1(dout) -> pol1_out1/1
  pol1_in1_last_tap/2(sync_out) -> sync_out/1
  pol1_in2/1 -> pol1_in2_first_tap/1(din)
  pol1_in2_first_tap/1(dout) -> pol1_in2_tap2/1
  pol1_in2_first_tap/2(sync_out) -> pol1_in2_tap2/2
  pol1_in2_first_tap/3(coeff_out) -> pol1_in2_tap2/3
  pol1_in2_first_tap/4(taps_out) -> pol1_in2_tap2/4
  pol1_in2_tap2/1 -> pol1_in2_tap3/1
  pol1_in2_tap2/2 -> pol1_in2_tap3/2
  pol1_in2_tap2/3 -> pol1_in2_tap3/3
  pol1_in2_tap2/4 -> pol1_in2_tap3/4
  pol1_in2_tap3/1 -> pol1_in2_last_tap/1(din)
  pol1_in2_tap3/2 -> pol1_in2_last_tap/2(sync)
  pol1_in2_tap3/3 -> pol1_in2_last_tap/3(coeff)
  pol1_in2_tap3/4 -> pol1_in2_last_tap/4(taps)
  pol1_in2_last_tap/1(dout) -> pol1_out2/1
  pol1_in3/1 -> pol1_in3_first_tap/1(din)
  pol1_in3_first_tap/1(dout) -> pol1_in3_tap2/1
  pol1_in3_first_tap/2(sync_out) -> pol1_in3_tap2/2
  pol1_in3_first_tap/3(coeff_out) -> pol1_in3_tap2/3
  pol1_in3_first_tap/4(taps_out) -> pol1_in3_tap2/4
  pol1_in3_tap2/1 -> pol1_in3_tap3/1
  pol1_in3_tap2/2 -> pol1_in3_tap3/2
  pol1_in3_tap2/3 -> pol1_in3_tap3/3
  pol1_in3_tap2/4 -> pol1_in3_tap3/4
  pol1_in3_tap3/1 -> pol1_in3_last_tap/1(din)
  pol1_in3_tap3/2 -> pol1_in3_last_tap/2(sync)
  pol1_in3_tap3/3 -> pol1_in3_last_tap/3(coeff)
  pol1_in3_tap3/4 -> pol1_in3_last_tap/4(taps)
  pol1_in3_last_tap/1(dout) -> pol1_out3/1
  pol1_in4/1 -> pol1_in4_first_tap/1(din)
  pol1_in4_first_tap/1(dout) -> pol1_in4_tap2/1
  pol1_in4_first_tap/2(sync_out) -> pol1_in4_tap2/2
  pol1_in4_first_tap/3(coeff_out) -> pol1_in4_tap2/3
  pol1_in4_first_tap/4(taps_out) -> pol1_in4_tap2/4
  pol1_in4_tap2/1 -> pol1_in4_tap3/1
  pol1_in4_tap2/2 -> pol1_in4_tap3/2
  pol1_in4_tap2/3 -> pol1_in4_tap3/3
  pol1_in4_tap2/4 -> pol1_in4_tap3/4
  pol1_in4_tap3/1 -> pol1_in4_last_tap/1(din)
  pol1_in4_tap3/2 -> pol1_in4_last_tap/2(sync)
  pol1_in4_tap3/3 -> pol1_in4_last_tap/3(coeff)
  pol1_in4_tap3/4 -> pol1_in4_last_tap/4(taps)
  pol1_in4_last_tap/1(dout) -> pol1_out4/1
  pol1_in5/1 -> pol1_in5_first_tap/1(din)
  pol1_in5_first_tap/1(dout) -> pol1_in5_tap2/1
  pol1_in5_first_tap/2(sync_out) -> pol1_in5_tap2/2
  pol1_in5_first_tap/3(coeff_out) -> pol1_in5_tap2/3
  pol1_in5_first_tap/4(taps_out) -> pol1_in5_tap2/4
  pol1_in5_tap2/1 -> pol1_in5_tap3/1
  pol1_in5_tap2/2 -> pol1_in5_tap3/2
  pol1_in5_tap2/3 -> pol1_in5_tap3/3
  pol1_in5_tap2/4 -> pol1_in5_tap3/4
  pol1_in5_tap3/1 -> pol1_in5_last_tap/1(din)
  pol1_in5_tap3/2 -> pol1_in5_last_tap/2(sync)
  pol1_in5_tap3/3 -> pol1_in5_last_tap/3(coeff)
  pol1_in5_tap3/4 -> pol1_in5_last_tap/4(taps)
  pol1_in5_last_tap/1(dout) -> pol1_out5/1
  pol1_in6/1 -> pol1_in6_first_tap/1(din)
  pol1_in6_first_tap/1(dout) -> pol1_in6_tap2/1
  pol1_in6_first_tap/2(sync_out) -> pol1_in6_tap2/2
  pol1_in6_first_tap/3(coeff_out) -> pol1_in6_tap2/3
  pol1_in6_first_tap/4(taps_out) -> pol1_in6_tap2/4
  pol1_in6_tap2/1 -> pol1_in6_tap3/1
  pol1_in6_tap2/2 -> pol1_in6_tap3/2
  pol1_in6_tap2/3 -> pol1_in6_tap3/3
  pol1_in6_tap2/4 -> pol1_in6_tap3/4
  pol1_in6_tap3/1 -> pol1_in6_last_tap/1(din)
  pol1_in6_tap3/2 -> pol1_in6_last_tap/2(sync)
  pol1_in6_tap3/3 -> pol1_in6_last_tap/3(coeff)
  pol1_in6_tap3/4 -> pol1_in6_last_tap/4(taps)
  pol1_in6_last_tap/1(dout) -> pol1_out6/1
  pol1_in7/1 -> pol1_in7_first_tap/1(din)
  pol1_in7_first_tap/1(dout) -> pol1_in7_tap2/1
  pol1_in7_first_tap/2(sync_out) -> pol1_in7_tap2/2
  pol1_in7_first_tap/3(coeff_out) -> pol1_in7_tap2/3
  pol1_in7_first_tap/4(taps_out) -> pol1_in7_tap2/4
  pol1_in7_tap2/1 -> pol1_in7_tap3/1
  pol1_in7_tap2/2 -> pol1_in7_tap3/2
  pol1_in7_tap2/3 -> pol1_in7_tap3/3
  pol1_in7_tap2/4 -> pol1_in7_tap3/4
  pol1_in7_tap3/1 -> pol1_in7_last_tap/1(din)
  pol1_in7_tap3/2 -> pol1_in7_last_tap/2(sync)
  pol1_in7_tap3/3 -> pol1_in7_last_tap/3(coeff)
  pol1_in7_tap3/4 -> pol1_in7_last_tap/4(taps)
  pol1_in7_last_tap/1(dout) -> pol1_out7/1
  pol1_in8/1 -> pol1_in8_first_tap/1(din)
  pol1_in8_first_tap/1(dout) -> pol1_in8_tap2/1
  pol1_in8_first_tap/2(sync_out) -> pol1_in8_tap2/2
  pol1_in8_first_tap/3(coeff_out) -> pol1_in8_tap2/3
  pol1_in8_first_tap/4(taps_out) -> pol1_in8_tap2/4
  pol1_in8_tap2/1 -> pol1_in8_tap3/1
  pol1_in8_tap2/2 -> pol1_in8_tap3/2
  pol1_in8_tap2/3 -> pol1_in8_tap3/3
  pol1_in8_tap2/4 -> pol1_in8_tap3/4
  pol1_in8_tap3/1 -> pol1_in8_last_tap/1(din)
  pol1_in8_tap3/2 -> pol1_in8_last_tap/2(sync)
  pol1_in8_tap3/3 -> pol1_in8_last_tap/3(coeff)
  pol1_in8_tap3/4 -> pol1_in8_last_tap/4(taps)
  pol1_in8_last_tap/1(dout) -> pol1_out8/1
  pol1_in9/1 -> pol1_in9_first_tap/1(din)
  pol1_in9_first_tap/1(dout) -> pol1_in9_tap2/1
  pol1_in9_first_tap/2(sync_out) -> pol1_in9_tap2/2
  pol1_in9_first_tap/3(coeff_out) -> pol1_in9_tap2/3
  pol1_in9_first_tap/4(taps_out) -> pol1_in9_tap2/4
  pol1_in9_tap2/1 -> pol1_in9_tap3/1
  pol1_in9_tap2/2 -> pol1_in9_tap3/2
  pol1_in9_tap2/3 -> pol1_in9_tap3/3
  pol1_in9_tap2/4 -> pol1_in9_tap3/4
  pol1_in9_tap3/1 -> pol1_in9_last_tap/1(din)
  pol1_in9_tap3/2 -> pol1_in9_last_tap/2(sync)
  pol1_in9_tap3/3 -> pol1_in9_last_tap/3(coeff)
  pol1_in9_tap3/4 -> pol1_in9_last_tap/4(taps)
  pol1_in9_last_tap/1(dout) -> pol1_out9/1
  pol1_in10/1 -> pol1_in10_first_tap/1(din)
  pol1_in10_first_tap/1(dout) -> pol1_in10_tap2/1
  pol1_in10_first_tap/2(sync_out) -> pol1_in10_tap2/2
  pol1_in10_first_tap/3(coeff_out) -> pol1_in10_tap2/3
  pol1_in10_first_tap/4(taps_out) -> pol1_in10_tap2/4
  pol1_in10_tap2/1 -> pol1_in10_tap3/1
  pol1_in10_tap2/2 -> pol1_in10_tap3/2
  pol1_in10_tap2/3 -> pol1_in10_tap3/3
  pol1_in10_tap2/4 -> pol1_in10_tap3/4
  pol1_in10_tap3/1 -> pol1_in10_last_tap/1(din)
  pol1_in10_tap3/2 -> pol1_in10_last_tap/2(sync)
  pol1_in10_tap3/3 -> pol1_in10_last_tap/3(coeff)
  pol1_in10_tap3/4 -> pol1_in10_last_tap/4(taps)
  pol1_in10_last_tap/1(dout) -> pol1_out10/1
  pol1_in11/1 -> pol1_in11_first_tap/1(din)
  pol1_in11_first_tap/1(dout) -> pol1_in11_tap2/1
  pol1_in11_first_tap/2(sync_out) -> pol1_in11_tap2/2
  pol1_in11_first_tap/3(coeff_out) -> pol1_in11_tap2/3
  pol1_in11_first_tap/4(taps_out) -> pol1_in11_tap2/4
  pol1_in11_tap2/1 -> pol1_in11_tap3/1
  pol1_in11_tap2/2 -> pol1_in11_tap3/2
  pol1_in11_tap2/3 -> pol1_in11_tap3/3
  pol1_in11_tap2/4 -> pol1_in11_tap3/4
  pol1_in11_tap3/1 -> pol1_in11_last_tap/1(din)
  pol1_in11_tap3/2 -> pol1_in11_last_tap/2(sync)
  pol1_in11_tap3/3 -> pol1_in11_last_tap/3(coeff)
  pol1_in11_tap3/4 -> pol1_in11_last_tap/4(taps)
  pol1_in11_last_tap/1(dout) -> pol1_out11/1
  pol1_in12/1 -> pol1_in12_first_tap/1(din)
  pol1_in12_first_tap/1(dout) -> pol1_in12_tap2/1
  pol1_in12_first_tap/2(sync_out) -> pol1_in12_tap2/2
  pol1_in12_first_tap/3(coeff_out) -> pol1_in12_tap2/3
  pol1_in12_first_tap/4(taps_out) -> pol1_in12_tap2/4
  pol1_in12_tap2/1 -> pol1_in12_tap3/1
  pol1_in12_tap2/2 -> pol1_in12_tap3/2
  pol1_in12_tap2/3 -> pol1_in12_tap3/3
  pol1_in12_tap2/4 -> pol1_in12_tap3/4
  pol1_in12_tap3/1 -> pol1_in12_last_tap/1(din)
  pol1_in12_tap3/2 -> pol1_in12_last_tap/2(sync)
  pol1_in12_tap3/3 -> pol1_in12_last_tap/3(coeff)
  pol1_in12_tap3/4 -> pol1_in12_last_tap/4(taps)
  pol1_in12_last_tap/1(dout) -> pol1_out12/1
  pol1_in13/1 -> pol1_in13_first_tap/1(din)
  pol1_in13_first_tap/1(dout) -> pol1_in13_tap2/1
  pol1_in13_first_tap/2(sync_out) -> pol1_in13_tap2/2
  pol1_in13_first_tap/3(coeff_out) -> pol1_in13_tap2/3
  pol1_in13_first_tap/4(taps_out) -> pol1_in13_tap2/4
  pol1_in13_tap2/1 -> pol1_in13_tap3/1
  pol1_in13_tap2/2 -> pol1_in13_tap3/2
  pol1_in13_tap2/3 -> pol1_in13_tap3/3
  pol1_in13_tap2/4 -> pol1_in13_tap3/4
  pol1_in13_tap3/1 -> pol1_in13_last_tap/1(din)
  pol1_in13_tap3/2 -> pol1_in13_last_tap/2(sync)
  pol1_in13_tap3/3 -> pol1_in13_last_tap/3(coeff)
  pol1_in13_tap3/4 -> pol1_in13_last_tap/4(taps)
  pol1_in13_last_tap/1(dout) -> pol1_out13/1
  pol1_in14/1 -> pol1_in14_first_tap/1(din)
  pol1_in14_first_tap/1(dout) -> pol1_in14_tap2/1
  pol1_in14_first_tap/2(sync_out) -> pol1_in14_tap2/2
  pol1_in14_first_tap/3(coeff_out) -> pol1_in14_tap2/3
  pol1_in14_first_tap/4(taps_out) -> pol1_in14_tap2/4
  pol1_in14_tap2/1 -> pol1_in14_tap3/1
  pol1_in14_tap2/2 -> pol1_in14_tap3/2
  pol1_in14_tap2/3 -> pol1_in14_tap3/3
  pol1_in14_tap2/4 -> pol1_in14_tap3/4
  pol1_in14_tap3/1 -> pol1_in14_last_tap/1(din)
  pol1_in14_tap3/2 -> pol1_in14_last_tap/2(sync)
  pol1_in14_tap3/3 -> pol1_in14_last_tap/3(coeff)
  pol1_in14_tap3/4 -> pol1_in14_last_tap/4(taps)
  pol1_in14_last_tap/1(dout) -> pol1_out14/1
  pol1_in15/1 -> pol1_in15_first_tap/1(din)
  pol1_in15_first_tap/1(dout) -> pol1_in15_tap2/1
  pol1_in15_first_tap/2(sync_out) -> pol1_in15_tap2/2
  pol1_in15_first_tap/3(coeff_out) -> pol1_in15_tap2/3
  pol1_in15_first_tap/4(taps_out) -> pol1_in15_tap2/4
  pol1_in15_tap2/1 -> pol1_in15_tap3/1
  pol1_in15_tap2/2 -> pol1_in15_tap3/2
  pol1_in15_tap2/3 -> pol1_in15_tap3/3
  pol1_in15_tap2/4 -> pol1_in15_tap3/4
  pol1_in15_tap3/1 -> pol1_in15_last_tap/1(din)
  pol1_in15_tap3/2 -> pol1_in15_last_tap/2(sync)
  pol1_in15_tap3/3 -> pol1_in15_last_tap/3(coeff)
  pol1_in15_tap3/4 -> pol1_in15_last_tap/4(taps)
  pol1_in15_last_tap/1(dout) -> pol1_out15/1
  pol1_in16/1 -> pol1_in16_first_tap/1(din)
  pol1_in16_first_tap/1(dout) -> pol1_in16_tap2/1
  pol1_in16_first_tap/2(sync_out) -> pol1_in16_tap2/2
  pol1_in16_first_tap/3(coeff_out) -> pol1_in16_tap2/3
  pol1_in16_first_tap/4(taps_out) -> pol1_in16_tap2/4
  pol1_in16_tap2/1 -> pol1_in16_tap3/1
  pol1_in16_tap2/2 -> pol1_in16_tap3/2
  pol1_in16_tap2/3 -> pol1_in16_tap3/3
  pol1_in16_tap2/4 -> pol1_in16_tap3/4
  pol1_in16_tap3/1 -> pol1_in16_last_tap/1(din)
  pol1_in16_tap3/2 -> pol1_in16_last_tap/2(sync)
  pol1_in16_tap3/3 -> pol1_in16_last_tap/3(coeff)
  pol1_in16_tap3/4 -> pol1_in16_last_tap/4(taps)
  pol1_in16_last_tap/1(dout) -> pol1_out16/1
